simulink model "TosAeb"  (R2021a)
settings: Solver=FixedStepDiscrete  StartTime=0.0  StopTime=inf  FixedStep=0.01  RelTol=1e-3
undefined symbols (not defined in the file or in its folder): ACCModSts, AccSts1, AEB_ObjectMovingOrientation, AEB_ObjectMovingState, CN_BTCActive, CN_CurrentGearR, CN_CurrentGearR_DHT, CN_PTCActive, CN_VDCActive, CN_VSC_EspOff, CN_VSC_FCW_AEB_Error, CN_VSC_FCW_AEB_NoDispay, CN_VSC_FCW_AEB_PerfmDgdtn, CN_VSC_FCW_AEB_TempUnAvlbl, CN_VSC_VehStandstill, CNaccelAvoidMax, CNaccelAvoidMin, CNbrakePedalPress, CNcomfortStrLeftAccelMax, CNcomfortStrLeftAccelMin, CNcomfortStrRightAccelMax, CNcomfortStrRightAccelMin, CNDrvDoorOpen, CNDrvModeEco, CNDrvModeNorm, CNDrvModeOther, CNDrvModeSport, CNDrvSBRreminder, CNNull, CNobjInvalidID, CNPassDoorOpen, CNPassSBRreminder, CNPEBSAccelNegInf, CNPEBSAccelNull, CNPEBSTimeInf, CNPEBSTimeNull, CNSTOPMAX, CNSTOPNULL, CNsubjWidth, CNSwtOFF, CNSwtON, CNTTCMAX, CNTTCNULL, CNVehConfigAEBCycNone, CNVehConfigAEBNone, CNVehConfigAEBPedNone, CNVehConfigFCWNone, CNVehConfigJANone, CollisionSeverity, FALSE, FCWSnvty, JACycObjCalcInfoDefaultParams, JAPedObjCalcInfoDefaultParams, JAVehObjCalcInfoDefaultParams, latCycObjCalcInfoDefaultParams, latPedObjCalcInfoDefaultParams, longObjCalcInfoDefaultParams, ModSts, movingStatus, ObjectType ... (+1112)
libraries: simulink x3031, AEBSFunctionLib x50, Ta_Library x33, AEBSStateMachineLib x23, Mylibrary x12, simulink_extras x7, ObjSelect_lib x3, dspstat3 x2
(part 47 of 102)

== system TosAeb/FcwAebJa_Step_sys/ALG_AEBSystem/ALG_AEBController/AEB_FuncImpManagementApp/FuncManeuArbCalc/brkFuncManeuArbCalc/brkManeuAborCalc/VelReduceMaxCond ==
  AEBTrigger : Inport
  subjVel : Inport  Port=2  OutDataTypeStr=single
  EBAFuncSts : Inport  Port=3
  P_FIM_AEBVelDecMaxThres : Constant  Value=P_AEB_FIM_AEBVelDecMaxThres
  P_FIM_EBAVelDecMaxThres : Constant  Value=P_AEB_FIM_EBAVelDecMaxThres
  vxTriggerCalc : Stateflow chart  TreatAsAtomicUnit=on
  VelReduceMaxCond : Outport
  wires:
  P_FIM_EBAVelDecMaxThres/1 -> vxTriggerCalc/4(P_FIM_EBAVelDecMaxThres)
  P_FIM_AEBVelDecMaxThres/1 -> vxTriggerCalc/5(P_FIM_AEBVelDecMaxThres)
  vxTriggerCalc/1(VelReduceMaxCond) -> VelReduceMaxCond/1
  EBAFuncSts/1 -> vxTriggerCalc/3(EBAFuncSts)
  AEBTrigger/1 -> vxTriggerCalc/1(AEBTrigger)
  subjVel/1 -> vxTriggerCalc/2(subjVel)

== system TosAeb/FcwAebJa_Step_sys/ALG_AEBSystem/ALG_AEBController/AEB_FuncImpManagementApp/FuncManeuArbCalc/brkFuncManeuArbCalc/brkManeuAborCalc/vehBrkManeuAborCalc ==
  AEBTrigger : Inport
  VelReduceMaxCond : Inport  Port=2
  dgrAborCond : Inport  Port=3
  ESPRelAborCond : Inport  Port=4
  velAborCond : Inport  Port=5
  Compare To Constant{,1..3} (4 alike) : simulink/Logic and Bit Operations/Compare To Constant  relop===  const=TRUE  OutDataTypeStr=boolean
  Logical Operator : Logic  Operator=OR  Inputs=4  AllPortsSameDT=off  OutDataTypeStr=boolean
  Logical Operator1 : Logic  AllPortsSameDT=off  OutDataTypeStr=boolean
  vehBrkManeuAbor : Outport
  wires:
  Compare To Constant3/1 -> Logical Operator/4
  velAborCond/1 -> Compare To Constant3/1
  Logical Operator1/1 -> vehBrkManeuAbor/1
  AEBTrigger/1 -> Logical Operator1/1
  Logical Operator/1 -> Logical Operator1/2
  Compare To Constant2/1 -> Logical Operator/3
  Compare To Constant1/1 -> Logical Operator/2
  Compare To Constant/1 -> Logical Operator/1
  ESPRelAborCond/1 -> Compare To Constant2/1
  dgrAborCond/1 -> Compare To Constant1/1
  VelReduceMaxCond/1 -> Compare To Constant/1

== system TosAeb/FcwAebJa_Step_sys/ALG_AEBSystem/ALG_AEBController/AEB_JaObjCalcApp/JAPedTgtInfoCalc/JAPedCollisonAssessInfo/LatPedCollisonAssessInfoCalc/PedDistBrakeFullCalc ==
  subjLongAccel : Inport  OutDataTypeStr=single
  subjVel : Inport  Port=2  OutDataTypeStr=single
  BrkSys : Subsystem
  Bus Creator : BusCreator  Inputs=6
  Bus Selector : BusSelector  OutputSignals=subjVel,tDelay,subjLongAccelFilt
  Bus Selector1 : BusSelector  OutputSignals=subjVel,Kjerk,tDelay,subjLongAccelFilt,accelMax
  Bus Selector2 : BusSelector  OutputSignals=tDelay,velDelta,subjVel,subjLongAccelFilt,Kjerk,accelMax,tDelay
  DistPhase1Calc : Subsystem
  DistPhase2Calc : Subsystem
  DistPhase3Calc : Subsystem
  distBrakeCalc : Subsystem
  tBrakeCalc : Subsystem
  distBrake : Outport
  tBrake : Outport  Port=2
  wires:
  DistPhase2Calc/2(egoStopFlg) -> DistPhase3Calc/1(egoStopFlg)
  DistPhase2Calc/3(tJerkWork) -> DistPhase3Calc/2(tJerkWork)
  DistPhase2Calc/4(tPhase2) -> [tPhase2], DistPhase3Calc/3(tPhase2)
  DistPhase3Calc/1(distPhase3) -> distBrakeCalc/3(distPhase3)
  DistPhase1Calc/1(distPhase1) -> distBrakeCalc/1(distPhase1)
  DistPhase2Calc/1(distPhase2) -> distBrakeCalc/2(distPhase2)
  distBrakeCalc/1(distBrake) -> distBrake/1
  subjVel/1 -> BrkSys/1(subjVel), Bus Creator/1  "subjVel"
  BrkSys/3(velDelta) -> Bus Creator/4  "velDelta"
  BrkSys/4(accelMax) -> Bus Creator/5  "accelMax"
  BrkSys/2(Kjerk) -> Bus Creator/3  "Kjerk"
  BrkSys/1(tDelay) -> Bus Creator/2  "tDelay"
  BrkSys/5(subjLongAccelFilt) -> Bus Creator/6  "subjLongAccelFilt"
  Bus Creator/1 -> Bus Selector2/1, Bus Selector1/1, Bus Selector/1
  Bus Selector/3 -> DistPhase1Calc/3(subjLongAccelFilt)  "<subjLongAccelFilt>"
  Bus Selector/2 -> DistPhase1Calc/2(tPhase1)  "<tDelay>"
  Bus Selector/1 -> DistPhase1Calc/1(subjVel)  "<subjVel>"
  Bus Selector1/4 -> DistPhase2Calc/4(subjLongAccelFilt)  "<subjLongAccelFilt>"
  Bus Selector1/2 -> DistPhase2Calc/2(Kjerk)  "<Kjerk>"
  Bus Selector1/1 -> DistPhase2Calc/1(subjVel)  "<subjVel>"
  Bus Selector1/5 -> DistPhase2Calc/5(accelMax)  "<accelMax>"
  Bus Selector2/1 -> DistPhase3Calc/4(tPhase1)  "<tDelay>"
  Bus Selector2/2 -> DistPhase3Calc/5(velDelta)  "<velDelta>"
  Bus Selector2/3 -> DistPhase3Calc/6(subjVel)  "<subjVel>"
  Bus Selector2/4 -> DistPhase3Calc/7(subjLongAccelFilt)  "<subjLongAccelFilt>"
  Bus Selector2/5 -> DistPhase3Calc/8(Kjerk)  "<Kjerk>"
  Bus Selector2/6 -> DistPhase3Calc/9(accelMax)  "<accelMax>"
  subjLongAccel/1 -> BrkSys/2(subjLongAccel)
  DistPhase3Calc/2(tPhase3) -> tBrakeCalc/1(tPhase3)
  Bus Selector2/7 -> tBrakeCalc/2(tPhase1)  "<tDelay>"
  [tPhase2] -> tBrakeCalc/3(tPhase2)
  tBrakeCalc/1(tBrake) -> tBrake/1
  Bus Selector1/3 -> DistPhase2Calc/3(tPhase1)  "<tDelay>"

== system TosAeb/FcwAebJa_Step_sys/ALG_AEBSystem/ALG_AEBController/AEB_JaObjCalcApp/JAPedTgtInfoCalc/JAPedCollisonAssessInfo/LatPedCollisonAssessInfoCalc/Subsystem1 ==
  vxAbsTargetCorr : Inport  OutDataTypeStr=single
  vyAbsTargetCorr : Inport  Port=2  OutDataTypeStr=single
  Trigonometric Function : Trigonometry  Operator=atan2
  TgtYawAngle : Outport
  wires:
  Trigonometric Function/1 -> TgtYawAngle/1
  vyAbsTargetCorr/1 -> Trigonometric Function/1
  vxAbsTargetCorr/1 -> Trigonometric Function/2

== system TosAeb/FcwAebJa_Step_sys/ALG_AEBSystem/ALG_AEBController/AEB_JaObjCalcApp/JAPedTgtInfoCalc/JAPedCollisonAssessInfo/LatPedCollisonAssessInfoCalc/VelAbsTgtCorrCalc ==
  vx : Inport  OutDataTypeStr=single
  vy : Inport  Port=2  OutDataTypeStr=single
  dy : Inport  Port=3  OutDataTypeStr=single
  dxFrontBumper : Inport  Port=4  OutDataTypeStr=single
  subjYawRate : Inport  Port=5  OutDataTypeStr=single
  subjVel : Inport  Port=6  OutDataTypeStr=single
  Bus Creator1 : BusCreator  Inputs=2
  RadicalRelevantCalc : Subsystem
  RadicalToXYTrans : Subsystem
  XYToRadicalTrans : Subsystem
  vxAbsTgtCorr : Outport
  vyAbsTgtCorr : Outport  Port=2
  wires:
  subjYawRate/1 -> RadicalRelevantCalc/3(subjYawRate)
  vx/1 -> Bus Creator1/1  "vx"
  dy/1 -> RadicalRelevantCalc/1(dy)
  RadicalToXYTrans/2(vyAbsTargetCorr) -> vyAbsTgtCorr/1
  RadicalRelevantCalc/2(sincosCoef) -> XYToRadicalTrans/3(sincosCoef), RadicalToXYTrans/2(sincosCoef)
  XYToRadicalTrans/1(velRadicalXY) -> RadicalToXYTrans/1(velRadicalXY)
  RadicalToXYTrans/1(vxAbsTargetCorr) -> vxAbsTgtCorr/1
  RadicalRelevantCalc/1(radialVelRel) -> XYToRadicalTrans/2(radialVelRel)  "radialVelRel"
  subjVel/1 -> RadicalToXYTrans/3(subjVel)
  Bus Creator1/1 -> XYToRadicalTrans/1(LongLatVelRel)  "LongLatVelRel"
  dxFrontBumper/1 -> RadicalRelevantCalc/2(dxFrontBumper)
  vy/1 -> Bus Creator1/2  "vy"

== system TosAeb/FcwAebJa_Step_sys/ALG_AEBSystem/ALG_AEBController/AEB_JaObjCalcApp/JAPedTgtInfoCalc/JAPedCollisonAssessInfo/LatPedCollisonAssessInfoCalc/distRadical2CollsionCalc ==
  vxAbsTgtCorr : Inport  OutDataTypeStr=single
  vyAbsTgtCorr : Inport  Port=2  OutDataTypeStr=single
  dx : Inport  Port=3  OutDataTypeStr=single
  dy : Inport  Port=4  OutDataTypeStr=single
  tPredictTime : Inport  Port=5  OutDataTypeStr=single
  Add : Sum  OutDataTypeStr=single
  Add1 : Sum  OutDataTypeStr=single
  Math Function : Math  Operator=hypot  SignedPower=on  SaturateOnIntegerOverflow=on
  Product : Product  OutDataTypeStr=single
  Product1 : Product  OutDataTypeStr=single
  distRadical2Collsion : Outport
  wires:
  vxAbsTgtCorr/1 -> Product/1
  tPredictTime/1 -> Product1/1, Product/2
  Product/1 -> Add/2
  dx/1 -> Add/1
  vyAbsTgtCorr/1 -> Product1/2
  Product1/1 -> Add1/1
  dy/1 -> Add1/2
  Add1/1 -> Math Function/2  "dyCollision"
  Add/1 -> Math Function/1  "dxCollision"
  Math Function/1 -> distRadical2Collsion/1  "distRadical"

== system TosAeb/FcwAebJa_Step_sys/ALG_AEBSystem/ALG_AEBController/AEB_JaObjCalcApp/JAPedTgtInfoCalc/JAPedCollisonAssessInfo/LatPedCollisonAssessInfoCalc/tBrakeingCalc ==
  note: 1) Add Subsystem or Model blocks as valid variant choices.
      | 2) You cannot connect blocks at this level. At simulation, connectivity is automatically
      | determined, based on the active variant and port name matching.
  tBarke : Inport  OutDataTypeStr=single
  subjVel : Inport  Port=2  OutDataTypeStr=single
  tBrakeCalc : Subsystem  VariantControl=(default)
  tBrakeLookup : Subsystem  VariantControl=tBrakeConfig == true
  TTB : Outport

== system TosAeb/FcwAebJa_Step_sys/ALG_AEBSystem/ALG_AEBController/AEB_JaObjCalcApp/JAPedTgtInfoCalc/JAPedObjCalcInfoCalc/JAPedObjInfoCalc/ObjCrossFlgCal ==
  AEBJAPedObj : Inport
  ObjAbsInfo : Inport  Port=2
  subjInfo : Inport  Port=3
  Bus Creator : BusCreator  Inputs=2  OutDataTypeStr=Bus: BusAEBJAPedObjColliInfo
      settings: InheritFromInputs=off
  Bus Selector : BusSelector  OutputSignals=subjVel,subjLongAccel,subjLatAccel,subjYawRate
  Bus Selector1 : BusSelector  OutputSignals=ObjectMovingOrientation,ObjectLength,ObjectTypeCameraConfirmed,ObjectWidth,dx,dy,ObjectID
  Bus Selector2 : BusSelector  OutputSignals=vyRelCorr,vxAbsObj,ObjectYawAngleCorr
  CollisionProbCalc : Subsystem
  CollisionProbCalcFlow : Stateflow chart  TreatAsAtomicUnit=on
  Constant2 : Constant  Value=P_AEB_JaPedCA_PreCollisionStepTime  OutDataTypeStr=single
  Constant3 : Constant  Value=P_AEB_JaPedCA_PreCollisionPredTimeMax  OutDataTypeStr=single
  ObjColliInfo : Outport
  wires:
  CollisionProbCalcFlow/2(tPredictTimeOut) -> CollisionProbCalc/1(tPredTime)
  CollisionProbCalcFlow/3(CollisionProbFunc()) -> [CrossFlg]
  Constant3/1 -> CollisionProbCalcFlow/2(P_JaPedCA_PreCollisionPredTimeMax)
  Constant2/1 -> CollisionProbCalcFlow/1(P_JaPedCA_PreCollisionStepTime)
  [CrossFlg] -> CollisionProbCalc/Trigger
  subjInfo/1 -> Bus Selector/1
  Bus Selector/1 -> CollisionProbCalc/2(subjVel)  "<subjVel>"
  Bus Selector/4 -> CollisionProbCalc/5(subjYawRate)  "<subjYawRate>"
  AEBJAPedObj/1 -> Bus Selector1/1
  ObjAbsInfo/1 -> Bus Selector2/1
  Bus Selector2/2 -> CollisionProbCalc/14(vxAbsObj)  "<vxAbsObj>"
  Bus Selector1/4 -> CollisionProbCalc/9(ObjectWidth)  "<ObjectWidth>"
  Bus Selector1/3 -> CollisionProbCalc/8(ObjectTypeCameraConfirmed)  "<ObjectTypeCameraConfirmed>"
  Bus Selector1/5 -> CollisionProbCalc/10(dx)  "<dx>"
  Bus Selector1/2 -> CollisionProbCalc/7(ObjectLength)  "<ObjectLength>"
  Bus Creator/1 -> ObjColliInfo/1
  CollisionProbCalc/1(ObjColliFlg) -> Bus Creator/2, CollisionProbCalcFlow/3(preCollisionProb)  "ObjColliFlg"
  Bus Selector1/6 -> CollisionProbCalc/11(dy)  "<dy>"
  Bus Selector1/7 -> CollisionProbCalc/12(ObjectID)  "<ObjectID>"
  Bus Selector2/1 -> CollisionProbCalc/13(vyRelCorr)  "<vyRelCorr>"
  Bus Selector1/1 -> CollisionProbCalc/6(ObjectMovingOrientation)  "<ObjectMovingOrientation>"
  Bus Selector/2 -> CollisionProbCalc/3(subjLongAccel)  "<subjLongAccel>"
  Bus Selector/3 -> CollisionProbCalc/4(subjLatAccel)  "<subjLatAccel>"
  CollisionProbCalcFlow/1(TTC) -> Bus Creator/1  "tPredictTime"
  Bus Selector2/3 -> CollisionProbCalc/15(ObjectYawAngleCorr)  "<ObjectYawAngleCorr>"

== system TosAeb/FcwAebJa_Step_sys/ALG_AEBSystem/ALG_AEBController/AEB_JaObjCalcApp/JAPedTgtInfoCalc/JAPedObjCalcInfoCalc/JAPedObjInfoCalc/SensorTgtInfo2AbsTgtInfoTrans ==
  dx : Inport  OutDataTypeStr=single
  dy : Inport  Port=2
  vx : Inport  Port=3  OutDataTypeStr=single
  ax : Inport  Port=4  OutDataTypeStr=single
  ObjectID : Inport  Port=5
  ObjectTypeCameraConfirmed : Inport  Port=6
  FR_ObjectID : Inport  Port=7
  FR_Dx : Inport  Port=8
  FR_Vy : Inport  Port=9
  FC_ObjectID : Inport  Port=10
  FC_Dx : Inport  Port=11
  FC_Vy : Inport  Port=12
  subjLongAccel : Inport  Port=13  OutDataTypeStr=single
  subjYawRate : Inport  Port=14  OutDataTypeStr=single
  subjVel : Inport  Port=15  OutDataTypeStr=single
  Constant : Constant  Value=P_Global_DxvFrontBumper_m  OutDataTypeStr=single
  Constant1 : Constant  Value=0  OutDataTypeStr=single
  Constant2 : Constant  Value=P_Global_EQ2RearAxisX_m  OutDataTypeStr=single
  FC_Dx1 : InportShadow  Port=11
  FR_Dx1 : InportShadow  Port=8
  Gain : Gain  Gain=0.5
  MinMax : MinMax  Function=max  Inputs=2
  ObjFusQualityCal : Stateflow chart  TreatAsAtomicUnit=on
  Product1 : Product  OutDataTypeStr=single
  Product2 : Product  OutDataTypeStr=single
  Product3 : Product  OutDataTypeStr=single
  Subtract : Sum  Inputs=+-  OutDataTypeStr=single
  Subtract{1..4,6..7} (6 alike) : Sum  OutDataTypeStr=single
  Subtract5 : Sum  Inputs=+-  OutDataTypeStr=single
  Trigonometric Function : Trigonometry  Operator=atan2
  dxFrontBumper : Outport
  vxAbsObj : Outport  Port=2
  axAbsObj : Outport  Port=3
  vyRelCorr : Outport  Port=4
  ObjFusQuality : Outport  Port=5
  ObjectYawAngleCorr : Outport  Port=6
  wires:
  subjVel/1 -> ObjFusQualityCal/7(subjVel), Subtract1/1
  FC_Dx/1 -> ObjFusQualityCal/6(FC_Dx)
  ObjectID/1 -> ObjFusQualityCal/1(ObjectID)
  ObjFusQualityCal/1(ObjFusQuality) -> ObjFusQuality/1
  ObjectTypeCameraConfirmed/1 -> ObjFusQualityCal/2(ObjectTypeCameraConfirmed)
  FR_ObjectID/1 -> ObjFusQualityCal/4(FR_ObjectID)
  FR_Dx/1 -> ObjFusQualityCal/5(FR_Dx)
  FC_ObjectID/1 -> ObjFusQualityCal/3(FC_ObjectID)
  Subtract/1 -> MinMax/1
  Constant1/1 -> MinMax/2
  subjYawRate/1 -> Product3/1, Product2/2, Product1/2
  Subtract3/1 -> Gain/1
  Constant/1 -> Subtract/2
  Subtract2/1 -> axAbsObj/1
  subjLongAccel/1 -> Subtract2/2
  ax/1 -> Subtract2/1
  Subtract1/1 -> Trigonometric Function/2, vxAbsObj/1
  MinMax/1 -> dxFrontBumper/1
  dx/1 -> Subtract/1
  Product1/1 -> Subtract4/2
  Subtract4/1 -> Subtract1/2
  vx/1 -> Subtract4/1
  dy/1 -> Product1/1
  Constant2/1 -> Subtract5/2
  FC_Dx1/1 -> Subtract5/1
  FR_Dx1/1 -> Product2/1
  Subtract5/1 -> Product3/2
  Product2/1 -> Subtract6/2
  FR_Vy/1 -> Subtract6/1
  Product3/1 -> Subtract7/2
  FC_Vy/1 -> Subtract7/1
  Subtract6/1 -> Subtract3/1
  Subtract7/1 -> Subtract3/2
  Trigonometric Function/1 -> ObjectYawAngleCorr/1
  Gain/1 -> Trigonometric Function/1, vyRelCorr/1

== system TosAeb/FcwAebJa_Step_sys/ALG_AEBSystem/ALG_AEBController/AEB_JaObjCalcApp/JAPedTgtInfoCalc/JAPedObjCalcInfoCalc/ObjPreSelectCondCalc/ObjIsInvalidCalc ==
  ObjectID : Inport
  Compare To Constant : simulink/Logic and Bit Operations/Compare To Constant  relop===  const=CNobjInvalidID  OutDataTypeStr=boolean
  ObjIsInvalid : Outport
  wires:
  Compare To Constant/1 -> ObjIsInvalid/1
  ObjectID/1 -> Compare To Constant/1

== system TosAeb/FcwAebJa_Step_sys/ALG_AEBSystem/ALG_AEBController/AEB_JaObjCalcApp/JAPedTgtInfoCalc/JAPedObjCalcInfoCalc/ObjPreSelectCondCalc/TgtPreSelectCondCalc ==
  note: change <= to <
  ObjectExistProb : Inport
  ObjectYawAngle : Inport  Port=2
  ObjectTypeCameraComfirmed : Inport  Port=3
  ObjectType : Inport  Port=4
  dxVariance : Inport  Port=5
  dx : Inport  Port=6
  dy : Inport  Port=7
  Action Port : ActionPort  ActionPortLabel=else
  Abs2 : Abs
  Compare To Constant1 : simulink/Logic and Bit Operations/Compare To Constant  relop===  const=TRUE  OutDataTypeStr=boolean
  Compare To Constant10 : simulink/Logic and Bit Operations/Compare To Constant  relop===  const=ObjectTypeCopy.BICYCLE  OutDataTypeStr=boolean
  Compare To Constant2 : simulink/Logic and Bit Operations/Compare To Constant  relop=>  const=P_AEB_JaPedCA_PreSelectObjectExistProbThres  OutDataTypeStr=boolean
  Compare To Constant3 : simulink/Logic and Bit Operations/Compare To Constant  relop=<  const=P_AEB_JaPedCA_PreSelectDxVarThres  OutDataTypeStr=boolean
  Compare To Constant4 : simulink/Logic and Bit Operations/Compare To Constant  relop=<  const=P_AEB_JaPedCA_PreSelectDxThres  OutDataTypeStr=boolean
  Compare To Constant6 : simulink/Logic and Bit Operations/Compare To Constant  relop===  const=ObjectTypeCopy.PEDESTRIAN  OutDataTypeStr=boolean
  Compare To Constant7 : simulink/Logic and Bit Operations/Compare To Constant  relop=>=  const=P_AEB_JaPedCA_PreSelectObjYawAngleThres  OutDataTypeStr=boolean
  Compare To Constant8 : simulink/Logic and Bit Operations/Compare To Constant  relop=<  const=P_AEB_JaPedCA_PreSelectDyThres  OutDataTypeStr=boolean
  Constant : Constant  Value=TRUE
  Constant1 : Constant  Value=P_Global_DxvFrontBumper_m  OutDataTypeStr=single
  Constant2 : Constant  Value=0  OutDataTypeStr=single
  Logical Operator : Logic  Operator=OR  AllPortsSameDT=off  OutDataTypeStr=boolean
  Logical Operator3 : Logic  Inputs=7  AllPortsSameDT=off  OutDataTypeStr=boolean
  MinMax : MinMax  Function=max  Inputs=2
  Subtract : Sum  Inputs=+-  OutDataTypeStr=single
  Terminator : Terminator
  ObjPreSelectCondFufilled : Outport
  wires:
  Compare To Constant2/1 -> Logical Operator3/1
  ObjectType/1 -> Compare To Constant6/1, Compare To Constant10/1
  Logical Operator3/1 -> ObjPreSelectCondFufilled/1
  ObjectTypeCameraComfirmed/1 -> Compare To Constant1/1
  ObjectExistProb/1 -> Compare To Constant2/1
  Compare To Constant7/1 -> Terminator/1
  Abs2/1 -> Compare To Constant7/1
  ObjectYawAngle/1 -> Abs2/1
  dxVariance/1 -> Compare To Constant3/1
  dy/1 -> Compare To Constant8/1
  Constant/1 -> Logical Operator3/2
  Compare To Constant3/1 -> Logical Operator3/5
  Compare To Constant4/1 -> Logical Operator3/6
  Compare To Constant8/1 -> Logical Operator3/7
  Compare To Constant1/1 -> Logical Operator3/3
  Constant2/1 -> MinMax/2
  Subtract/1 -> MinMax/1
  Constant1/1 -> Subtract/2
  dx/1 -> Subtract/1
  MinMax/1 -> Compare To Constant4/1
  Compare To Constant10/1 -> Logical Operator/1
  Compare To Constant6/1 -> Logical Operator/2
  Logical Operator/1 -> Logical Operator3/4

== system TosAeb/FcwAebJa_Step_sys/ALG_AEBSystem/ALG_AEBController/AEB_JaObjCalcApp/JAPedTgtInfoCalc/JAPedObjCalcInfoCalc/ObjPreSelectCondCalc/TgtPreSelectCondCalc1 ==
  Action Port : ActionPort  ActionPortLabel=if(u1)
  Constant : Constant  Value=FALSE  OutDataTypeStr=boolean
  ObjPreSelectCondFufilled : Outport
  wires:
  Constant/1 -> ObjPreSelectCondFufilled/1

== system TosAeb/FcwAebJa_Step_sys/ALG_AEBSystem/ALG_AEBController/AEB_JaObjCalcApp/JAVehTgtInfoCalc/AEBJAVehObjCalc/JAVehObjInfoCalc/ObjCrossFlgCal ==
  BusAEBJAVehObj : Inport
  ObjAbsInfo : Inport  Port=2
  subjInfo : Inport  Port=3
  Bus Creator : BusCreator  Inputs=2  OutDataTypeStr=Bus: BusAEBJAVehObjColliInfo
  Bus Selector : BusSelector  OutputSignals=subjVel,subjLongAccel,subjLatAccel,subjYawRate
  Bus Selector1 : BusSelector  OutputSignals=ObjectYawAngle,ObjectMovingOrientation,ObjectLength,FusionType,ObjectWidth,dx,vy,ay,dy,ObjectID
  Bus Selector2 : BusSelector  OutputSignals=vxAbsObj,axAbsObj
  CollisionProbCalc : Subsystem
  CollisionProbCalcFlow : Stateflow chart  TreatAsAtomicUnit=on
  Constant2 : Constant  Value=P_AEB_JaVehCA_PreCollisionStepTime  OutDataTypeStr=single
  Constant3 : Constant  Value=P_AEB_JaVehCA_PreCollisionPredTimeMax  OutDataTypeStr=single
  ObjColliInfo : Outport
  wires:
  CollisionProbCalcFlow/2(tPredictTimeOut) -> CollisionProbCalc/1(tPredTime)
  CollisionProbCalcFlow/3(CollisionProbFunc()) -> [CrossFlg]
  Constant3/1 -> CollisionProbCalcFlow/2(P_JaVehCA_PreCollisionPredTimeMax)
  Constant2/1 -> CollisionProbCalcFlow/1(P_JaVehCA_PreCollisionStepTime)
  [CrossFlg] -> CollisionProbCalc/Trigger
  subjInfo/1 -> Bus Selector/1
  Bus Selector/1 -> CollisionProbCalc/2(subjVel)  "<subjVel>"
  Bus Selector/4 -> CollisionProbCalc/5(subjYawRate)  "<subjYawRate>"
  BusAEBJAVehObj/1 -> Bus Selector1/1
  ObjAbsInfo/1 -> Bus Selector2/1
  Bus Selector2/2 -> CollisionProbCalc/17(axAbsObj)  "<axAbsObj>"
  Bus Selector2/1 -> CollisionProbCalc/16(vxAbsObj)  "<vxAbsObj>"
  Bus Selector1/5 -> CollisionProbCalc/10(ObjectWidth)  "<ObjectWidth>"
  Bus Selector1/4 -> CollisionProbCalc/9(FusionType)  "<FusionType>"
  Bus Selector1/6 -> CollisionProbCalc/11(dx)  "<dx>"
  Bus Selector1/3 -> CollisionProbCalc/8(ObjectLength)  "<ObjectLength>"
  Bus Selector1/7 -> CollisionProbCalc/12(vy)  "<vy>"
  Bus Selector1/1 -> CollisionProbCalc/6(ObjectYawAngle)  "<ObjectYawAngle>"
  Bus Selector1/8 -> CollisionProbCalc/13(ay)  "<ay>"
  Bus Selector1/9 -> CollisionProbCalc/14(dy)  "<dy>"
  Bus Creator/1 -> ObjColliInfo/1
  Bus Selector1/10 -> CollisionProbCalc/15(ObjectID)  "<ObjectID>"
  CollisionProbCalc/1(ObjColliFlg) -> Bus Creator/2, CollisionProbCalcFlow/3(preCollisionProb)  "ObjColliFlg"
  Bus Selector1/2 -> CollisionProbCalc/7(ObjectMovingOrientation)  "<ObjectMovingOrientation>"
  Bus Selector/2 -> CollisionProbCalc/3(subjLongAccel)  "<subjLongAccel>"
  Bus Selector/3 -> CollisionProbCalc/4(subjLatAccel)  "<subjLatAccel>"
  CollisionProbCalcFlow/1(TTC) -> Bus Creator/1  "tPredictTime"

== system TosAeb/FcwAebJa_Step_sys/ALG_AEBSystem/ALG_AEBController/AEB_JaObjCalcApp/JAVehTgtInfoCalc/AEBJAVehObjCalc/JAVehObjInfoCalc/SensorTgtInfo2AbsTgtInfoTrans ==
  dx : Inport  OutDataTypeStr=single
  vx : Inport  Port=2  OutDataTypeStr=single
  ax : Inport  Port=3  OutDataTypeStr=single
  vy : Inport  Port=4  OutDataTypeStr=single
  ObjectID : Inport  Port=5
  ObjectTypeCameraConfirmed : Inport  Port=6
  FR_ObjectID : Inport  Port=7
  FR_Dx : Inport  Port=8
  FR_Dy : Inport  Port=9
  FC_ObjectID : Inport  Port=10
  FC_Dx : Inport  Port=11
  FC_Dy : Inport  Port=12
  subjVel : Inport  Port=13
  subjLongAccel : Inport  Port=14
  subjYawRate : Inport  Port=15
  Constant : Constant  Value=P_Global_DxvFrontBumper_m  OutDataTypeStr=single
  Constant1 : Constant  Value=0  OutDataTypeStr=single
  MinMax : MinMax  Function=max  Inputs=2
  ObjFusQualityCal : Stateflow chart  TreatAsAtomicUnit=on
  Product : Product  OutDataTypeStr=single
  Subtract : Sum  Inputs=+-  OutDataTypeStr=single
  Subtract1 : Sum  OutDataTypeStr=single
  Subtract2 : Sum  OutDataTypeStr=single
  Subtract3 : Sum  OutDataTypeStr=single
  dx1 : InportShadow  OutDataTypeStr=single
  dxFrontBumper : Outport
  vxAbsObj : Outport  Port=2
  axAbsObj : Outport  Port=3
  vyRelCorr : Outport  Port=4
  ObjFusQuality : Outport  Port=5
  wires:
  dx/1 -> Subtract/1
  MinMax/1 -> dxFrontBumper/1
  Subtract1/1 -> vxAbsObj/1
  ax/1 -> Subtract2/1
  subjLongAccel/1 -> Subtract2/2
  Subtract2/1 -> axAbsObj/1
  Constant/1 -> Subtract/2
  Subtract3/1 -> vyRelCorr/1
  Product/1 -> Subtract3/2
  vy/1 -> Subtract3/1
  dx1/1 -> Product/1
  subjYawRate/1 -> Product/2
  Constant1/1 -> MinMax/2
  Subtract/1 -> MinMax/1
  ObjFusQualityCal/1(ObjFusQuality) -> ObjFusQuality/1
  FC_Dy/1 -> ObjFusQualityCal/8(FC_Dy)
  ObjectTypeCameraConfirmed/1 -> ObjFusQualityCal/2(ObjectTypeCameraConfirmed)
  FC_Dx/1 -> ObjFusQualityCal/7(FC_Dx)
  FR_ObjectID/1 -> ObjFusQualityCal/4(FR_ObjectID)
  FR_Dx/1 -> ObjFusQualityCal/6(FR_Dx)
  FC_ObjectID/1 -> ObjFusQualityCal/3(FC_ObjectID)
  ObjectID/1 -> ObjFusQualityCal/1(ObjectID)
  FR_Dy/1 -> ObjFusQualityCal/5(FR_Dy)
  subjVel/1 -> Subtract1/1, ObjFusQualityCal/9(subjVel)
  vx/1 -> Subtract1/2

== system TosAeb/FcwAebJa_Step_sys/ALG_AEBSystem/ALG_AEBController/AEB_JaObjCalcApp/JAVehTgtInfoCalc/AEBJAVehObjCalc/ObjPreSelectCondCalc/ObjIsInvalidCalc ==
  ObjectID : Inport
  Compare To Constant : simulink/Logic and Bit Operations/Compare To Constant  relop===  const=CNobjInvalidID  OutDataTypeStr=boolean
  ObjIsInvalid : Outport
  wires:
  Compare To Constant/1 -> ObjIsInvalid/1
  ObjectID/1 -> Compare To Constant/1

== system TosAeb/FcwAebJa_Step_sys/ALG_AEBSystem/ALG_AEBController/AEB_JaObjCalcApp/JAVehTgtInfoCalc/AEBJAVehObjCalc/ObjPreSelectCondCalc/TgtPreSelect ==
  ObjectExistProb : Inport
  ObjectMovingState : Inport  Port=2
  ObjectMovingOrientation : Inport  Port=3
  ObjectTypeCameraConfirmed : Inport  Port=4
  ObjectType : Inport  Port=5
  dxVariance : Inport  Port=6
  vx : Inport  Port=7  OutDataTypeStr=single
  dy : Inport  Port=8
  dx : Inport  Port=9
  subjVel : Inport  Port=10
  Action Port : ActionPort  ActionPortLabel=else
  Abs : Abs
  Abs1 : Abs
  Compare To Constant : simulink/Logic and Bit Operations/Compare To Constant  relop=<  const=P_AEB_JaVehCA_PreSelectDyThres  OutDataTypeStr=boolean
  Compare To Constant1 : simulink/Logic and Bit Operations/Compare To Constant  relop===  const=TRUE  OutDataTypeStr=boolean
  Compare To Constant10 : simulink/Logic and Bit Operations/Compare To Constant  relop===  const=ObjectTypeCopy.CAR  OutDataTypeStr=boolean
  Compare To Constant11 : simulink/Logic and Bit Operations/Compare To Constant  relop===  const=ObjectTypeCopy.TRUCK  OutDataTypeStr=boolean
  Compare To Constant2 : simulink/Logic and Bit Operations/Compare To Constant  relop=>  const=P_AEB_JaVehCA_PreSelectObjectExistProbThres  OutDataTypeStr=boolean
  Compare To Constant3 : simulink/Logic and Bit Operations/Compare To Constant  relop=<  const=P_AEB_JaVehCA_PreSelectDxVarThres  OutDataTypeStr=boolean
  Compare To Constant4 : simulink/Logic and Bit Operations/Compare To Constant  relop=<=  const=P_AEB_JaVehCA_PreSelecVxAbsObjAbsThres  OutDataTypeStr=boolean
  Compare To Constant5 : simulink/Logic and Bit Operations/Compare To Constant  relop===  const=AEB_ObjectMovingState.Moving  OutDataTypeStr=boolean
  Compare To Constant6 : simulink/Logic and Bit Operations/Compare To Constant  relop=<  const=P_AEB_JaVehCA_PreSelectDxThres  OutDataTypeStr=boolean
  Compare To Constant7 : simulink/Logic and Bit Operations/Compare To Constant  relop===  const=AEB_ObjectMovingOrientation.InvDir  OutDataTypeStr=boolean
  Constant : Constant  Value=P_Global_DxvFrontBumper_m  OutDataTypeStr=single
  Constant1 : Constant  Value=0  OutDataTypeStr=single
  Logical Operator3 : Logic  Inputs=9  AllPortsSameDT=off  OutDataTypeStr=boolean
  Logical Operator4 : Logic  Operator=OR  AllPortsSameDT=off  OutDataTypeStr=boolean
  MinMax : MinMax  Function=max  Inputs=2
  Subtract : Sum  Inputs=+-  OutDataTypeStr=single
  Subtract1 : Sum  OutDataTypeStr=single
  ObjPreSelectCondFufilled : Outport  OutDataTypeStr=boolean
  wires:
  ObjectExistProb/1 -> Compare To Constant2/1
  dxVariance/1 -> Compare To Constant3/1
  ObjectMovingState/1 -> Compare To Constant5/1
  Logical Operator3/1 -> ObjPreSelectCondFufilled/1
  Compare To Constant2/1 -> Logical Operator3/1
  Compare To Constant3/1 -> Logical Operator3/6
  Compare To Constant4/1 -> Logical Operator3/7
  Compare To Constant5/1 -> Logical Operator3/2
  ObjectTypeCameraConfirmed/1 -> Compare To Constant1/1
  Compare To Constant1/1 -> Logical Operator3/4
  Logical Operator4/1 -> Logical Operator3/5
  Compare To Constant10/1 -> Logical Operator4/1
  Compare To Constant11/1 -> Logical Operator4/2
  ObjectType/1 -> Compare To Constant10/1, Compare To Constant11/1
  Compare To Constant7/1 -> Logical Operator3/3
  ObjectMovingOrientation/1 -> Compare To Constant7/1
  Abs1/1 -> Compare To Constant4/1
  Abs/1 -> Compare To Constant/1
  dy/1 -> Abs/1
  Compare To Constant/1 -> Logical Operator3/8
  Compare To Constant6/1 -> Logical Operator3/9
  Constant1/1 -> MinMax/2
  Constant/1 -> Subtract/2
  Subtract/1 -> MinMax/1
  dx/1 -> Subtract/1
  MinMax/1 -> Compare To Constant6/1
  vx/1 -> Subtract1/1
  subjVel/1 -> Subtract1/2
  Subtract1/1 -> Abs1/1

== system TosAeb/FcwAebJa_Step_sys/ALG_AEBSystem/ALG_AEBController/AEB_JaObjCalcApp/JAVehTgtInfoCalc/AEBJAVehObjCalc/ObjPreSelectCondCalc/TgtPreSelectCondDefault ==
  Action Port : ActionPort  ActionPortLabel=if(u1)
  Constant : Constant  Value=FALSE  OutDataTypeStr=boolean
  ObjPreSelectCondFufilled : Outport
  wires:
  Constant/1 -> ObjPreSelectCondFufilled/1

== system TosAeb/FcwAebJa_Step_sys/ALG_AEBSystem/ALG_AEBController/AEB_JaObjCalcApp/JAVehTgtInfoCalc/JAVehCollisonAssessInfoCalc/JaVehCollisonAssessInfoCalc/VehDistBrakeFullCalc ==
  subjLongAccel : Inport  OutDataTypeStr=single
  subjVel : Inport  Port=2  OutDataTypeStr=single
  BrkSys : Subsystem
  Bus Creator : BusCreator  Inputs=6
  Bus Selector : BusSelector  OutputSignals=subjVel,tDelay,subjLongAccelFilt
  Bus Selector1 : BusSelector  OutputSignals=subjVel,Kjerk,subjLongAccelFilt,accelMax
  Bus Selector2 : BusSelector  OutputSignals=tDelay,velDelta,subjVel,subjLongAccelFilt,Kjerk,accelMax,tDelay
  DistPhase1Calc : Subsystem
  DistPhase2Calc : Subsystem
  DistPhase3Calc : Subsystem
  distBrakeCalc : Subsystem
  tBrakeCalc : Subsystem
  distBrake : Outport
  tBrake : Outport  Port=2
  wires:
  DistPhase2Calc/2(egoStopFlg) -> DistPhase3Calc/1(egoStopFlg)
  DistPhase2Calc/3(tJerkWork) -> DistPhase3Calc/2(tJerkWork)
  DistPhase2Calc/4(tPhase2) -> [tPhase2], DistPhase3Calc/3(tPhase2)
  DistPhase3Calc/1(distPhase3) -> distBrakeCalc/3(distPhase3)
  DistPhase1Calc/1(distPhase1) -> distBrakeCalc/1(distPhase1)
  DistPhase2Calc/1(distPhase2) -> distBrakeCalc/2(distPhase2)
  distBrakeCalc/1(distBrake) -> distBrake/1
  subjVel/1 -> BrkSys/1(subjVel), Bus Creator/1  "subjVel"
  BrkSys/3(velDelta) -> Bus Creator/4  "velDelta"
  BrkSys/4(accelMax) -> Bus Creator/5  "accelMax"
  BrkSys/2(Kjerk) -> Bus Creator/3  "Kjerk"
  BrkSys/1(tDelay) -> Bus Creator/2  "tDelay"
  BrkSys/5(subjLongAccelFilt) -> Bus Creator/6  "subjLongAccelFilt"
  Bus Creator/1 -> Bus Selector2/1, Bus Selector1/1, Bus Selector/1
  Bus Selector/3 -> DistPhase1Calc/3(subjLongAccelFilt)  "<subjLongAccelFilt>"
  Bus Selector/2 -> DistPhase1Calc/2(tPhase1)  "<tDelay>"
  Bus Selector/1 -> DistPhase1Calc/1(subjVel)  "<subjVel>"
  Bus Selector1/3 -> DistPhase2Calc/3(subjLongAccelFilt)  "<subjLongAccelFilt>"
  Bus Selector1/2 -> DistPhase2Calc/2(Kjerk)  "<Kjerk>"
  Bus Selector1/1 -> DistPhase2Calc/1(subjVel)  "<subjVel>"
  Bus Selector1/4 -> DistPhase2Calc/4(accelMax)  "<accelMax>"
  Bus Selector2/1 -> DistPhase3Calc/4(tPhase1)  "<tDelay>"
  Bus Selector2/2 -> DistPhase3Calc/5(velDelta)  "<velDelta>"
  Bus Selector2/3 -> DistPhase3Calc/6(subjVel)  "<subjVel>"
  Bus Selector2/4 -> DistPhase3Calc/7(subjLongAccelFilt)  "<subjLongAccelFilt>"
  Bus Selector2/5 -> DistPhase3Calc/8(Kjerk)  "<Kjerk>"
  Bus Selector2/6 -> DistPhase3Calc/9(accelMax)  "<accelMax>"
  subjLongAccel/1 -> BrkSys/2(subjLongAccel)
  [tPhase2] -> tBrakeCalc/2(tPhase2)
  Bus Selector2/7 -> tBrakeCalc/3(tPhase1)  "<tDelay>"
  tBrakeCalc/1(tBrake) -> tBrake/1
  DistPhase3Calc/2(tPhase3) -> tBrakeCalc/1(tPhase3)

== system TosAeb/FcwAebJa_Step_sys/ALG_AEBSystem/ALG_AEBController/AEB_JaObjCalcApp/JAVehTgtInfoCalc/JAVehCollisonAssessInfoCalc/JaVehCollisonAssessInfoCalc/VelAbsTgtCorrCalc ==
  vx : Inport  OutDataTypeStr=single
  vy : Inport  Port=2  OutDataTypeStr=single
  dy : Inport  Port=3  OutDataTypeStr=single
  dxFrontBumper : Inport  Port=4  OutDataTypeStr=single
  subjYawRate : Inport  Port=5  OutDataTypeStr=single
  subjVel : Inport  Port=6  OutDataTypeStr=single
  Bus Creator1 : BusCreator  Inputs=2
  RadicalRelevantCalc : Subsystem
  RadicalToXYTrans : Subsystem
  XYToRadicalTrans : Subsystem
  vxAbsTgtCorr : Outport
  vyAbsTgtCorr : Outport  Port=2
  wires:
  subjYawRate/1 -> RadicalRelevantCalc/3(subjYawRate)
  vx/1 -> Bus Creator1/1  "vx"
  dy/1 -> RadicalRelevantCalc/1(dy)
  RadicalToXYTrans/2(vyAbsTargetCorr) -> vyAbsTgtCorr/1
  RadicalRelevantCalc/2(sincosCoef) -> XYToRadicalTrans/3(sincosCoef), RadicalToXYTrans/2(sincosCoef)
  XYToRadicalTrans/1(velRadicalXY) -> RadicalToXYTrans/1(velRadicalXY)
  RadicalToXYTrans/1(vxAbsTargetCorr) -> vxAbsTgtCorr/1
  RadicalRelevantCalc/1(radialVelRel) -> XYToRadicalTrans/2(radialVelRel)  "radialVelRel"
  subjVel/1 -> RadicalToXYTrans/3(subjVel)
  Bus Creator1/1 -> XYToRadicalTrans/1(LongLatVelRel)  "LongLatVelRel"
  dxFrontBumper/1 -> RadicalRelevantCalc/2(dxFrontBumper)
  vy/1 -> Bus Creator1/2  "vy"

== system TosAeb/FcwAebJa_Step_sys/ALG_AEBSystem/ALG_AEBController/AEB_JaObjCalcApp/JAVehTgtInfoCalc/JAVehCollisonAssessInfoCalc/JaVehCollisonAssessInfoCalc/distRadical2CollsionCalc ==
  vxAbsTgtCorr : Inport  OutDataTypeStr=single
  vyAbsTgtCorr : Inport  Port=2  OutDataTypeStr=single
  dx : Inport  Port=3  OutDataTypeStr=single
  tPredictTime : Inport  Port=4  OutDataTypeStr=single
  distBrake : Inport  Port=5  OutDataTypeStr=single
  Abs : Abs  OutDataTypeStr=single
  Add : Sum  OutDataTypeStr=single
  Add2 : Sum  Inputs=+-  OutDataTypeStr=single
  Product : Product  OutDataTypeStr=single
  Product2 : Product  OutDataTypeStr=single
  Product3 : Product  OutDataTypeStr=single
  Trigonometric Function : Trigonometry  Operator=atan2
  Trigonometric Function1 : Trigonometry
  initialLatDist : Outport
  restLatDist2Collsion : Outport  Port=2
  wires:
  tPredictTime/1 -> Product/1
  Product/1 -> Add/2
  Add/1 -> Product2/1  "dxCollision"
  vxAbsTgtCorr/1 -> Product/2, Trigonometric Function/2
  vyAbsTgtCorr/1 -> Trigonometric Function/1
  Trigonometric Function/1 -> Abs/1
  Trigonometric Function1/1 -> Product2/2, Product3/1  "TgtYawAngle"
  Add2/1 -> restLatDist2Collsion/1  "restLatDist2Collsion"
  distBrake/1 -> Product3/2
  Product2/1 -> initialLatDist/1, Add2/1
  Product3/1 -> Add2/2
  dx/1 -> Add/1
  Abs/1 -> Trigonometric Function1/1

== system TosAeb/FcwAebJa_Step_sys/ALG_AEBSystem/ALG_AEBController/AEB_JaObjCalcApp/JAVehTgtInfoCalc/JAVehCollisonAssessInfoCalc/JaVehCollisonAssessInfoCalc/tBrakeingCalc ==
  note: 1) Add Subsystem or Model blocks as valid variant choices.
      | 2) You cannot connect blocks at this level. At simulation, connectivity is automatically
      | determined, based on the active variant and port name matching.
  tBarke : Inport  OutDataTypeStr=single
  subjVel : Inport  Port=2  OutDataTypeStr=single
  tBrakeCalc : Subsystem  VariantControl=(default)
  tBrakeLookup : Subsystem  VariantControl=tBrakeConfig == true
  TTB : Outport

== system TosAeb/FcwAebJa_Step_sys/ALG_AEBSystem/ALG_AEBController/AEB_JaObjCalcApp/JaCycTgtInfoCalc/JaCycCollisonAssessInfo/JaCycCollisonAssessInfoCalc/PedDistBrakeFullCalc ==
  subjLongAccel : Inport  OutDataTypeStr=single
  subjVel : Inport  Port=2  OutDataTypeStr=single
  BrkSys : Subsystem
  Bus Creator : BusCreator  Inputs=6
  Bus Selector : BusSelector  OutputSignals=subjVel,tDelay,subjLongAccelFilt
  Bus Selector1 : BusSelector  OutputSignals=subjVel,Kjerk,tDelay,subjLongAccelFilt,accelMax
  Bus Selector2 : BusSelector  OutputSignals=tDelay,velDelta,subjVel,subjLongAccelFilt,Kjerk,accelMax,tDelay
  DistPhase1Calc : Subsystem
  DistPhase2Calc : Subsystem
  DistPhase3Calc : Subsystem
  distBrakeCalc : Subsystem
  tBrakeCalc : Subsystem
  distBrake : Outport
  tBrake : Outport  Port=2
  wires:
  DistPhase2Calc/2(egoStopFlg) -> DistPhase3Calc/1(egoStopFlg)
  DistPhase2Calc/3(tJerkWork) -> DistPhase3Calc/2(tJerkWork)
  DistPhase2Calc/4(tPhase2) -> [tPhase2], DistPhase3Calc/3(tPhase2)
  DistPhase3Calc/1(distPhase3) -> distBrakeCalc/3(distPhase3)
  DistPhase1Calc/1(distPhase1) -> distBrakeCalc/1(distPhase1)
  DistPhase2Calc/1(distPhase2) -> distBrakeCalc/2(distPhase2)
  distBrakeCalc/1(distBrake) -> distBrake/1
  subjVel/1 -> BrkSys/1(subjVel), Bus Creator/1  "subjVel"
  BrkSys/3(velDelta) -> Bus Creator/4  "velDelta"
  BrkSys/4(accelMax) -> Bus Creator/5  "accelMax"
  BrkSys/2(Kjerk) -> Bus Creator/3  "Kjerk"
  BrkSys/1(tDelay) -> Bus Creator/2  "tDelay"
  BrkSys/5(subjLongAccelFilt) -> Bus Creator/6  "subjLongAccelFilt"
  Bus Creator/1 -> Bus Selector2/1, Bus Selector1/1, Bus Selector/1
  Bus Selector/3 -> DistPhase1Calc/3(subjLongAccelFilt)  "<subjLongAccelFilt>"
  Bus Selector/2 -> DistPhase1Calc/2(tPhase1)  "<tDelay>"
  Bus Selector/1 -> DistPhase1Calc/1(subjVel)  "<subjVel>"
  Bus Selector1/4 -> DistPhase2Calc/4(subjLongAccelFilt)  "<subjLongAccelFilt>"
  Bus Selector1/2 -> DistPhase2Calc/2(Kjerk)  "<Kjerk>"
  Bus Selector1/1 -> DistPhase2Calc/1(subjVel)  "<subjVel>"
  Bus Selector1/5 -> DistPhase2Calc/5(accelMax)  "<accelMax>"
  Bus Selector2/1 -> DistPhase3Calc/4(tPhase1)  "<tDelay>"
  Bus Selector2/2 -> DistPhase3Calc/5(velDelta)  "<velDelta>"
  Bus Selector2/3 -> DistPhase3Calc/6(subjVel)  "<subjVel>"
  Bus Selector2/4 -> DistPhase3Calc/7(subjLongAccelFilt)  "<subjLongAccelFilt>"
  Bus Selector2/5 -> DistPhase3Calc/8(Kjerk)  "<Kjerk>"
  Bus Selector2/6 -> DistPhase3Calc/9(accelMax)  "<accelMax>"
  subjLongAccel/1 -> BrkSys/2(subjLongAccel)
  DistPhase3Calc/2(tPhase3) -> tBrakeCalc/1(tPhase3)
  Bus Selector2/7 -> tBrakeCalc/2(tPhase1)  "<tDelay>"
  [tPhase2] -> tBrakeCalc/3(tPhase2)
  tBrakeCalc/1(tBrake) -> tBrake/1
  Bus Selector1/3 -> DistPhase2Calc/3(tPhase1)  "<tDelay>"

== system TosAeb/FcwAebJa_Step_sys/ALG_AEBSystem/ALG_AEBController/AEB_JaObjCalcApp/JaCycTgtInfoCalc/JaCycCollisonAssessInfo/JaCycCollisonAssessInfoCalc/Subsystem1 ==
  vxAbsTargetCorr : Inport  OutDataTypeStr=single
  vyAbsTargetCorr : Inport  Port=2  OutDataTypeStr=single
  Trigonometric Function : Trigonometry  Operator=atan2
  TgtYawAngle : Outport
  wires:
  Trigonometric Function/1 -> TgtYawAngle/1
  vyAbsTargetCorr/1 -> Trigonometric Function/1
  vxAbsTargetCorr/1 -> Trigonometric Function/2

== system TosAeb/FcwAebJa_Step_sys/ALG_AEBSystem/ALG_AEBController/AEB_JaObjCalcApp/JaCycTgtInfoCalc/JaCycCollisonAssessInfo/JaCycCollisonAssessInfoCalc/VelAbsTgtCorrCalc ==
  vx : Inport  OutDataTypeStr=single
  vy : Inport  Port=2  OutDataTypeStr=single
  dy : Inport  Port=3  OutDataTypeStr=single
  dxFrontBumper : Inport  Port=4  OutDataTypeStr=single
  subjYawRate : Inport  Port=5  OutDataTypeStr=single
  subjVel : Inport  Port=6  OutDataTypeStr=single
  Bus Creator1 : BusCreator  Inputs=2
  RadicalRelevantCalc : Subsystem
  RadicalToXYTrans : Subsystem
  XYToRadicalTrans : Subsystem
  vxAbsTgtCorr : Outport
  vyAbsTgtCorr : Outport  Port=2
  wires:
  subjYawRate/1 -> RadicalRelevantCalc/3(subjYawRate)
  vx/1 -> Bus Creator1/1  "vx"
  dy/1 -> RadicalRelevantCalc/1(dy)
  RadicalToXYTrans/2(vyAbsTargetCorr) -> vyAbsTgtCorr/1
  RadicalRelevantCalc/2(sincosCoef) -> XYToRadicalTrans/3(sincosCoef), RadicalToXYTrans/2(sincosCoef)
  XYToRadicalTrans/1(velRadicalXY) -> RadicalToXYTrans/1(velRadicalXY)
  RadicalToXYTrans/1(vxAbsTargetCorr) -> vxAbsTgtCorr/1
  RadicalRelevantCalc/1(radialVelRel) -> XYToRadicalTrans/2(radialVelRel)  "radialVelRel"
  subjVel/1 -> RadicalToXYTrans/3(subjVel)
  Bus Creator1/1 -> XYToRadicalTrans/1(LongLatVelRel)  "LongLatVelRel"
  dxFrontBumper/1 -> RadicalRelevantCalc/2(dxFrontBumper)
  vy/1 -> Bus Creator1/2  "vy"

== system TosAeb/FcwAebJa_Step_sys/ALG_AEBSystem/ALG_AEBController/AEB_JaObjCalcApp/JaCycTgtInfoCalc/JaCycCollisonAssessInfo/JaCycCollisonAssessInfoCalc/distRadical2CollsionCalc ==
  vxAbsTgtCorr : Inport  OutDataTypeStr=single
  vyAbsTgtCorr : Inport  Port=2  OutDataTypeStr=single
  dx : Inport  Port=3  OutDataTypeStr=single
  dy : Inport  Port=4  OutDataTypeStr=single
  tPredictTime : Inport  Port=5  OutDataTypeStr=single
  Add : Sum  OutDataTypeStr=single
  Add1 : Sum  OutDataTypeStr=single
  Math Function : Math  Operator=hypot  SignedPower=on  SaturateOnIntegerOverflow=on
  Product : Product  OutDataTypeStr=single
  Product1 : Product  OutDataTypeStr=single
  distRadical2Collsion : Outport
  wires:
  vxAbsTgtCorr/1 -> Product/1
  tPredictTime/1 -> Product1/1, Product/2
  Product/1 -> Add/2
  dx/1 -> Add/1
  vyAbsTgtCorr/1 -> Product1/2
  Product1/1 -> Add1/1
  dy/1 -> Add1/2
  Add1/1 -> Math Function/2  "dyCollision"
  Add/1 -> Math Function/1  "dxCollision"
  Math Function/1 -> distRadical2Collsion/1  "distRadical"

== system TosAeb/FcwAebJa_Step_sys/ALG_AEBSystem/ALG_AEBController/AEB_JaObjCalcApp/JaCycTgtInfoCalc/JaCycObjCalcInfoCalc/JaCycObjInfoCalc/ObjCrossFlgCal ==
  JaCycObj : Inport
  ObjAbsInfo : Inport  Port=2
  subjInfo : Inport  Port=3
  Bus Creator : BusCreator  Inputs=2  OutDataTypeStr=Bus: BusAEBJaCycObjColliInfo
      settings: InheritFromInputs=off
  Bus Selector : BusSelector  OutputSignals=subjVel,subjLongAccel,subjLatAccel,subjYawRate
  Bus Selector1 : BusSelector  OutputSignals=ObjectTypeCameraConfirmed,ObjectLength,ObjectWidth,ObjectMovingOrientation,dx,dy,ObjectID
  Bus Selector2 : BusSelector  OutputSignals=vyRelCorr,vxAbsObj,ObjectYawAngleCorr
  CollisionProbCalc : Subsystem
  CollisionProbCalcFlow : Stateflow chart  TreatAsAtomicUnit=on
  Constant2 : Constant  Value=P_AEB_JaCycCA_PreCollisionStepTime  OutDataTypeStr=single
  Constant3 : Constant  Value=P_AEB_JaCycCA_PreCollisionPredTimeMax  OutDataTypeStr=single
  ObjColliInfo : Outport
  wires:
  CollisionProbCalcFlow/2(tPredictTimeOut) -> CollisionProbCalc/1(tPredTime)
  CollisionProbCalcFlow/3(CollisionProbFunc()) -> [CrossFlg]
  Constant3/1 -> CollisionProbCalcFlow/2(P_JaPedCA_PreCollisionPredTimeMax)
  Constant2/1 -> CollisionProbCalcFlow/1(P_JaPedCA_PreCollisionStepTime)
  [CrossFlg] -> CollisionProbCalc/Trigger
  subjInfo/1 -> Bus Selector/1
  Bus Selector/1 -> CollisionProbCalc/2(subjVel)  "<subjVel>"
  Bus Selector/4 -> CollisionProbCalc/5(subjYawRate)  "<subjYawRate>"
  JaCycObj/1 -> Bus Selector1/1
  ObjAbsInfo/1 -> Bus Selector2/1
  Bus Selector2/2 -> CollisionProbCalc/14(vxAbsObj)  "<vxAbsObj>"
  Bus Selector1/3 -> CollisionProbCalc/8(ObjectWidth)  "<ObjectWidth>"
  Bus Selector1/1 -> CollisionProbCalc/6(ObjectTypeCameraConfirmed)  "<ObjectTypeCameraConfirmed>"
  Bus Selector1/5 -> CollisionProbCalc/10(dx)  "<dx>"
  Bus Selector1/2 -> CollisionProbCalc/7(ObjectLength)  "<ObjectLength>"
  Bus Creator/1 -> ObjColliInfo/1
  CollisionProbCalc/1(ObjColliFlg) -> CollisionProbCalcFlow/3(preCollisionProb), Bus Creator/2  "ObjColliFlg"
  Bus Selector1/6 -> CollisionProbCalc/11(dy)  "<dy>"
  Bus Selector1/7 -> CollisionProbCalc/12(ObjectID)  "<ObjectID>"
  Bus Selector2/1 -> CollisionProbCalc/13(vyRelCorr)  "<vyRelCorr>"
  Bus Selector1/4 -> CollisionProbCalc/9(ObjectMovingOrientation)  "<ObjectMovingOrientation>"
  Bus Selector/2 -> CollisionProbCalc/3(subjLongAccel)  "<subjLongAccel>"
  Bus Selector/3 -> CollisionProbCalc/4(subjLatAccel)  "<subjLatAccel>"
  CollisionProbCalcFlow/1(TTC) -> Bus Creator/1  "tPredictTime"
  Bus Selector2/3 -> CollisionProbCalc/15(ObjectYawAngleCorr)  "<ObjectYawAngleCorr>"

== system TosAeb/FcwAebJa_Step_sys/ALG_AEBSystem/ALG_AEBController/AEB_JaObjCalcApp/JaCycTgtInfoCalc/JaCycObjCalcInfoCalc/JaCycObjInfoCalc/SensorTgtInfo2AbsTgtInfoTrans1 ==
  dx : Inport  OutDataTypeStr=single
  dy : Inport  Port=2
  vx : Inport  Port=3  OutDataTypeStr=single
  vy : Inport  Port=4
  ax : Inport  Port=5  OutDataTypeStr=single
  ObjectID : Inport  Port=6
  ObjectTypeCameraConfirmed : Inport  Port=7
  FR_ObjectID : Inport  Port=8
  FR_Dx : Inport  Port=9
  FR_Vy : Inport  Port=10
  FC_ObjectID : Inport  Port=11
  FC_Dx : Inport  Port=12
  FC_Vy : Inport  Port=13
  subjLongAccel : Inport  Port=14  OutDataTypeStr=single
  subjYawRate : Inport  Port=15  OutDataTypeStr=single
  subjVel : Inport  Port=16  OutDataTypeStr=single
  Constant : Constant  Value=P_Global_DxvFrontBumper_m  OutDataTypeStr=single
  Constant1 : Constant  Value=0  OutDataTypeStr=single
  Constant2 : Constant  Value=P_Global_EQ2RearAxisX_m  OutDataTypeStr=single
  FC_Dx1 : InportShadow  Port=12
  FR_Dx1 : InportShadow  Port=9
  Gain : Gain  Gain=0.5
  MinMax : MinMax  Function=max  Inputs=2
  ObjFusQualityCal : Stateflow chart  TreatAsAtomicUnit=on
  Product1 : Product  OutDataTypeStr=single
  Product2 : Product  OutDataTypeStr=single
  Product3 : Product  OutDataTypeStr=single
  Subtract : Sum  Inputs=+-  OutDataTypeStr=single
  Subtract{1..4,6..7} (6 alike) : Sum  OutDataTypeStr=single
  Subtract5 : Sum  Inputs=+-  OutDataTypeStr=single
  Terminator : Terminator
  Trigonometric Function : Trigonometry  Operator=atan2
  dxFrontBumper : Outport
  vxAbsObj : Outport  Port=2
  axAbsObj : Outport  Port=3
  vyRelCorr : Outport  Port=4
  ObjFusQuality : Outport  Port=5
  ObjectYawAngleCorr : Outport  Port=6
  wires:
  subjVel/1 -> ObjFusQualityCal/7(subjVel), Subtract1/1
  FC_Dx/1 -> ObjFusQualityCal/6(FC_Dx)
  ObjectID/1 -> ObjFusQualityCal/1(ObjectID)
  ObjFusQualityCal/1(ObjFusQuality) -> ObjFusQuality/1
  ObjectTypeCameraConfirmed/1 -> ObjFusQualityCal/2(ObjectTypeCameraConfirmed)
  FR_ObjectID/1 -> ObjFusQualityCal/4(FR_ObjectID)
  FR_Dx/1 -> ObjFusQualityCal/5(FR_Dx)
  FC_ObjectID/1 -> ObjFusQualityCal/3(FC_ObjectID)
  Subtract/1 -> MinMax/1
  Constant1/1 -> MinMax/2
  subjYawRate/1 -> Product3/1, Product2/2, Product1/2
  Subtract3/1 -> Gain/1
  Constant/1 -> Subtract/2
  Subtract2/1 -> axAbsObj/1
  subjLongAccel/1 -> Subtract2/2
  ax/1 -> Subtract2/1
  Subtract1/1 -> Trigonometric Function/2, vxAbsObj/1
  MinMax/1 -> dxFrontBumper/1
  dx/1 -> Subtract/1
  Product1/1 -> Subtract4/2
  Subtract4/1 -> Subtract1/2
  vx/1 -> Subtract4/1
  dy/1 -> Product1/1
  Constant2/1 -> Subtract5/2
  FC_Dx1/1 -> Subtract5/1
  FR_Dx1/1 -> Product2/1
  Subtract5/1 -> Product3/2
  Product2/1 -> Subtract6/2
  FR_Vy/1 -> Subtract6/1
  Product3/1 -> Subtract7/2
  FC_Vy/1 -> Subtract7/1
  Subtract6/1 -> Subtract3/1
  Subtract7/1 -> Subtract3/2
  vy/1 -> vyRelCorr/1, Trigonometric Function/1
  Trigonometric Function/1 -> ObjectYawAngleCorr/1
  Gain/1 -> Terminator/1

== system TosAeb/FcwAebJa_Step_sys/ALG_AEBSystem/ALG_AEBController/AEB_JaObjCalcApp/JaCycTgtInfoCalc/JaCycObjCalcInfoCalc/ObjPreSelectCondCalc/ObjIsInvalidCalc ==
  ObjectID : Inport
  Compare To Constant : simulink/Logic and Bit Operations/Compare To Constant  relop===  const=CNobjInvalidID  OutDataTypeStr=boolean
  ObjIsInvalid : Outport
  wires:
  Compare To Constant/1 -> ObjIsInvalid/1
  ObjectID/1 -> Compare To Constant/1

== system TosAeb/FcwAebJa_Step_sys/ALG_AEBSystem/ALG_AEBController/AEB_JaObjCalcApp/JaCycTgtInfoCalc/JaCycObjCalcInfoCalc/ObjPreSelectCondCalc/TgtPreSelectCondCalc ==
  note: change <= to <
  ObjectExistProb : Inport
  ObjectYawAngle : Inport  Port=2
  ObjectTypeCameraComfirmed : Inport  Port=3
  ObjectType : Inport  Port=4
  dxVariance : Inport  Port=5
  dx : Inport  Port=6
  dy : Inport  Port=7
  Action Port : ActionPort  ActionPortLabel=else
  Abs2 : Abs
  Compare To Constant1 : simulink/Logic and Bit Operations/Compare To Constant  relop===  const=TRUE  OutDataTypeStr=boolean
  Compare To Constant10 : simulink/Logic and Bit Operations/Compare To Constant  relop===  const=ObjectTypeCopy.BICYCLE  OutDataTypeStr=boolean
  Compare To Constant2 : simulink/Logic and Bit Operations/Compare To Constant  relop=>  const=P_AEB_JaCycCA_PreSelectObjectExistProbThres  OutDataTypeStr=boolean
  Compare To Constant3 : simulink/Logic and Bit Operations/Compare To Constant  relop=<  const=P_AEB_JaCycCA_PreSelectDxVarThres  OutDataTypeStr=boolean
  Compare To Constant4 : simulink/Logic and Bit Operations/Compare To Constant  relop=<  const=P_AEB_JaCycCA_PreSelectDxThres  OutDataTypeStr=boolean
  Compare To Constant6 : simulink/Logic and Bit Operations/Compare To Constant  relop===  const=ObjectTypeCopy.MOTORBIKE  OutDataTypeStr=boolean
  Compare To Constant7 : simulink/Logic and Bit Operations/Compare To Constant  relop=>=  const=P_AEB_JaCycCA_PreSelectObjYawAngleThres  OutDataTypeStr=boolean
  Compare To Constant8 : simulink/Logic and Bit Operations/Compare To Constant  relop=<  const=P_AEB_JaCycCA_PreSelectDyThres  OutDataTypeStr=boolean
  Constant : Constant  Value=TRUE
  Constant1 : Constant  Value=P_Global_DxvFrontBumper_m  OutDataTypeStr=single
  Constant2 : Constant  Value=0  OutDataTypeStr=single
  Logical Operator : Logic  Operator=OR  AllPortsSameDT=off  OutDataTypeStr=boolean
  Logical Operator3 : Logic  Inputs=7  AllPortsSameDT=off  OutDataTypeStr=boolean
  MinMax : MinMax  Function=max  Inputs=2
  Subtract : Sum  Inputs=+-  OutDataTypeStr=single
  Terminator : Terminator
  ObjPreSelectCondFufilled : Outport
  wires:
  Compare To Constant6/1 -> Logical Operator/2
  Compare To Constant10/1 -> Logical Operator/1
  Logical Operator/1 -> Logical Operator3/4
  Compare To Constant2/1 -> Logical Operator3/1
  ObjectType/1 -> Compare To Constant6/1, Compare To Constant10/1
  Logical Operator3/1 -> ObjPreSelectCondFufilled/1
  ObjectTypeCameraComfirmed/1 -> Compare To Constant1/1
  ObjectExistProb/1 -> Compare To Constant2/1
  Compare To Constant7/1 -> Terminator/1
  Abs2/1 -> Compare To Constant7/1
  ObjectYawAngle/1 -> Abs2/1
  dxVariance/1 -> Compare To Constant3/1
  dy/1 -> Compare To Constant8/1
  Constant/1 -> Logical Operator3/2
  Compare To Constant3/1 -> Logical Operator3/5
  Compare To Constant4/1 -> Logical Operator3/6
  Compare To Constant8/1 -> Logical Operator3/7
  Compare To Constant1/1 -> Logical Operator3/3
  Constant2/1 -> MinMax/2
  Subtract/1 -> MinMax/1
  Constant1/1 -> Subtract/2
  dx/1 -> Subtract/1
  MinMax/1 -> Compare To Constant4/1

== system TosAeb/FcwAebJa_Step_sys/ALG_AEBSystem/ALG_AEBController/AEB_JaObjCalcApp/JaCycTgtInfoCalc/JaCycObjCalcInfoCalc/ObjPreSelectCondCalc/TgtPreSelectCondCalc1 ==
  Action Port : ActionPort  ActionPortLabel=if(u1)
  Constant : Constant  Value=FALSE  OutDataTypeStr=boolean
  ObjPreSelectCondFufilled : Outport
  wires:
  Constant/1 -> ObjPreSelectCondFufilled/1
